simulink model "control"  (R2020b)
settings: Solver=VariableStepAuto  StartTime=0.0  StopTime=20  FixedStep=0.0001  RelTol=1e-3
PreLoadFcn:
  | load('data.mat');
model workspace (MAT data in the model file):
  LUT = [0.000510726493303 0.00051026523397 0.000510216027351 0.00051017496753 0.000510239529035 0.000510188586418 0.000510178918773 0.000510209816127 0.000510206521938 0.000510199701921 0.000510191190244 0.000510212888059 ... (5994000 elements, 999x6000)]
  V0step = 0.1
  apogee = 700
  force = 710
  k_max = 0.01
  kstep = 0.005
undefined symbols (not defined in the file or in its folder): m
libraries: pid_lib x1
(part 1 of 2)
settings Model: OrderedModelArguments=off  Creator=Lucas  LastModifiedBy=Lucas
settings DebuggingCC: UnderSpecifiedDimensionMsg=warning

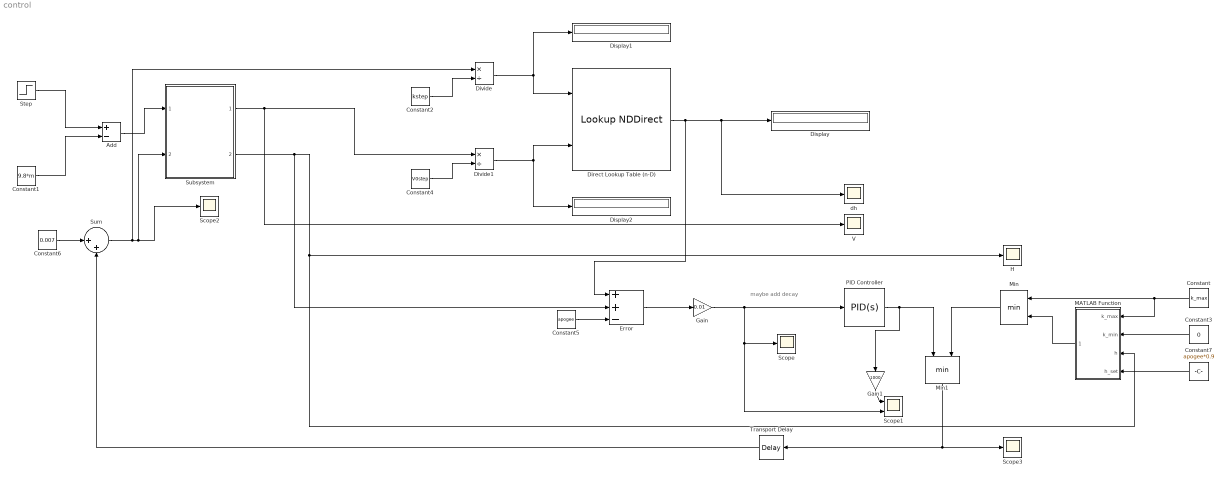
== system control ==
  note: maybe add decay
  Add : Sum  Inputs=+-
  Constant : Constant  Value=k_max
  Constant1 : Constant  Value=9.8*m
  Constant2 : Constant  Value=kstep
  Constant3 : Constant  Value=0
  Constant4 : Constant  Value=V0step
  Constant5 : Constant  Value=apogee
  Constant6 : Constant  Value=0.007
  Constant7 : Constant  Value=apogee*0.9
  Direct Lookup Table (n-D) : LookupNDDirect  Table=LUT
  Display : Display
  Display1 : Display
  Display2 : Display
  Divide : Product  Inputs=*/
  Divide1 : Product  Inputs=*/
  Error : Sum  Inputs=++-
  Gain : Gain  Gain=0.01
  Gain1 : Gain  Gain=1000
  H : Scope
  MATLAB Function : MATLAB Function  TreatAsAtomicUnit=on
  Min : MinMax  Inputs=2
  Min1 : MinMax  Inputs=2
  PID Controller : pid_lib/PID Controller  Controller=PID  Form=Parallel  TimeDomain=Continuous-time  UseExternalTs=off  SampleTime=-1  IntegratorMethod=Forward Euler  FilterMethod=Forward Euler  ControllerParametersSource=internal  P=0.01  I=-0.0001  D=0.001  UseFilter=on  N=3  TunerSelectOption=Frequency Response Based  InitialConditionSource=internal  InitialConditionForIntegrator=0  InitialConditionForFilter=0  DifferentiatorICPrevScaledInput=0  ExternalReset=none  TrackingMode=off  Kt=1  LimitOutput=on  SatLimitsSource=internal  UpperSaturationLimit=0.01  LowerSaturationLimit=0  LinearizeAsGain=off  AntiWindupMode=none  Kb=1  POutMin=[]  POutMax=[]  IOutMin=[]  IOutMax=[]  DOutMin=[]  DOutMax=[]  NOutMin=[]  NOutMax=[]  SumOutMin=[]  SumOutMax=[]  SaturationOutMin=[]  SaturationOutMax=[]  PParamMin=[]  PParamMax=[]  IParamMin=[]  IParamMax=[]  DParamMin=[]  DParamMax=[]  NParamMin=[]  NParamMax=[]  KbParamMin=[]  KbParamMax=[]  KtParamMin=[]  KtParamMax=[]  KbOutMin=[]  KbOutMax=[]  KtOutMin=[]  KtOutMax=[]  IntegratorOutMin=[]  IntegratorOutMax=[]  FilterOutMin=[]  FilterOutMax=[]  SumI1OutMin=[]  SumI1OutMax=[]  SumI2OutMin=[]  SumI2OutMax=[]  SumI3OutMin=[]  SumI3OutMax=[]  SumI4OutMin=[]  SumI4OutMax=[]  SumDOutMin=[]  SumDOutMax=[]  DifferentiatorOutMin=[]  DifferentiatorOutMax=[]  FilterDiffOutCoefMin=[]  FilterDiffOutCoefMax=[]  ReciprocalOutMin=[]  ReciprocalOutMax=[]  SumDenOutMin=[]  SumDenOutMax=[]  SumNumOutMin=[]  SumNumOutMax=[]  DivideOutMin=[]  DivideOutMax=[]  UdiffTsProdOutMin=[]  UdiffTsProdOutMax=[]  NTsProdOutMin=[]  NTsProdOutMax=[]  UintegralTsProdOutMin=[]  UintegralTsProdOutMax=[]  UngainTsProdOutMin=[]  UngainTsProdOutMax=[]  IntegratorContinuousStateAttributes=''  IntegratorStateMustResolveToSignalObject=off  IntegratorRTWStateStorageClass=Auto  FilterContinuousStateAttributes=''  FilterStateMustResolveToSignalObject=off  FilterRTWStateStorageClass=Auto  ParallelPVariant=InternalParameters  IdealPVariant=Disabled  IVariant=InternalParameters  DVariant=InternalParameters  IntegratorVariant=Continuous  SatVariant=Enabled  AWVariant=Passthrough  PCopyVariant=Disabled  TRVariant=Disabled  FdbkBlocksVariant=Forward  IdealPFdbkVariant=Disabled  SatFdbkVariant=Disabled  DerivativeFilterVariant=ContFilter  NVariant=InternalParameters  NCopyVariant=Disabled  FilterICVariant=InternalICsFilter  IntegratorICVariant=InternalICs  ExternalResetVariant=Disabled  TRSumVariant=Passthrough  SumFdbkVariant=Disabled  SumVariant=Sum_PID  TsampFilterVariant=InternalTs  TsampNgainVariant=Passthrough  TsampIntegralVariant=Passthrough  ExternalSaturationOutDataTypeStr=Inherit: Same as second input
  Scope : Scope
  Scope1 : Scope  NumInputPorts=2
  Scope2 : Scope
  Scope3 : Scope
  Step : Step  Time=8  Before=force  After=0  SampleTime=0
  Subsystem : Subsystem  ReferencedSubsystem=rocket_rough
  Sum : Sum  Inputs=|++
  Transport Delay : TransportDelay  DelayTime=0.05
  V : Scope
  dh : Scope
  wires:
  Direct Lookup Table (n-D)/1 -> dh/1, Display/1, Error/1
  Constant2/1 -> Divide/2
  Divide/1 -> Direct Lookup Table (n-D)/1, Display1/1
  Constant4/1 -> Divide1/2
  Divide1/1 -> Direct Lookup Table (n-D)/2, Display2/1
  Add/1 -> Subsystem/1
  Step/1 -> Add/1
  Constant1/1 -> Add/2
  Constant5/1 -> Error/3
  Constant6/1 -> Sum/1
  Sum/1 -> Scope2/1, Subsystem/2, Divide/1
  Subsystem/1 -> V/1, Divide1/1
  Subsystem/2 -> MATLAB Function/3(h), H/1, Error/2
  Transport Delay/1 -> Sum/2
  MATLAB Function/1(k_comp) -> Min/2
  Constant/1 -> MATLAB Function/1(k_max), Min/1
  Constant3/1 -> MATLAB Function/2(k_min)
  Constant7/1 -> MATLAB Function/4(h_set)
  Min1/1 -> Transport Delay/1, Scope3/1
  Gain1/1 -> Scope1/1
  Error/1 -> Gain/1
  Min/1 -> Min1/2
  PID Controller/1 -> Min1/1, Gain1/1
  Gain/1 -> Scope1/2, Scope/1, PID Controller/1
